simulink model "v01_aa_ri_8r4t11f_ver102"  (Simulink 7.6)
settings: Solver=ode45  StartTime=0.0  StopTime=128000  RelTol=1e-3
libraries: xbsIndex_r4 x13461, monroe_library x80, xbsReplacements_r4 x42, xps_library x36, casper_library_misc x35, simulink x23, dspsigops x16
(part 9 of 99)

== system v01_aa_ri_8r4t11f_ver102/adcsnap_b/bram_msb ==
  addr : Inport
  data_in : Inport  Port=2
  we : Inport  Port=3
  calc_add : Subsystem
  convert_addr : xbsIndex_r4/Convert  gui_display_data_type=Fixed-point  arith_type=Unsigned  n_bits=addr_width  bin_pt=0  float_type=Single  exp_bits=8  fraction_bits=24  quantization=Truncate  overflow=Wrap  en=off  latency=0  dbl_ovrd=off  pipeline=off  block_type=convert
      settings: xl_area=[0, 0, 0, 0, 0, 0, 0]
  convert_din : xbsIndex_r4/Convert  gui_display_data_type=Fixed-point  arith_type=Unsigned  n_bits=data_width  bin_pt=data_bin_pt  float_type=Single  exp_bits=8  fraction_bits=24  quantization=Truncate  overflow=Wrap  en=off  latency=0  dbl_ovrd=off  pipeline=off  block_type=convert
      settings: xl_area=[0, 0, 0, 0, 0, 0, 0]
  convert_din1 : xbsIndex_r4/Convert  gui_display_data_type=Fixed-point  arith_type=Unsigned  n_bits=data_width  bin_pt=data_bin_pt  float_type=Single  exp_bits=8  fraction_bits=24  quantization=Truncate  overflow=Wrap  en=off  latency=0  dbl_ovrd=off  pipeline=off  block_type=convert
      settings: xl_area=[0, 0, 0, 0, 0, 0, 0]
  convert_we : xbsIndex_r4/Convert  gui_display_data_type=Fixed-point  arith_type=Unsigned  n_bits=1  bin_pt=0  float_type=Single  exp_bits=8  fraction_bits=24  quantization=Truncate  overflow=Wrap  en=off  latency=0  dbl_ovrd=off  pipeline=off  block_type=convert
      settings: xl_area=[0, 0, 0, 0, 0, 0, 0]
  mem : Subsystem
  munge_in : Subsystem  AncestorBlock=casper_library_flow_control/munge  mask(munge){divisions=1, div_size=32, order=[0]}
      mask prompts: divisions "Number of divisions"; div_size "Division size (bits)"; order "Division packing order"
      mask help: Splits an input bus into the specified number of divisions and then recombines them in the order specified (most significant division = 0,least = number divisions-1)
      mask init:
      | munge_init(gcb, ...
      |     'divisions', divisions, ...
      |     'div_size', div_size, ...
      |     'order', order);
      settings: AttributesFormatString=|
      | [0]
      | 32 bits
  munge_out : Subsystem  AncestorBlock=casper_library_flow_control/munge  mask(munge){divisions=1, div_size=32, order=[0]}
      mask prompts: divisions "Number of divisions"; div_size "Division size (bits)"; order "Division packing order"
      mask help: Splits an input bus into the specified number of divisions and then recombines them in the order specified (most significant division = 0,least = number divisions-1)
      mask init:
      | munge_init(gcb, ...
      |     'divisions', divisions, ...
      |     'div_size', div_size, ...
      |     'order', order);
      settings: AttributesFormatString=|
      | [0]
      | 32 bits
  v01_aa_ri_8r4t11f_ver102_adcsnap_b_bram_msb_addr : xbsIndex_r4/Gateway Out  hdl_port=on  timing_constraint=None  locs_specified=off  LOCs={'A5','B9','A9','C1','A3','G6','E9','A6','A7','A8','B8','B1','B3','D9','B5','B4','B6','C2'}  block_type=gatewayout
      settings: infoedit="Gateway out block.  Converts Xilinx fixed point inputs into ouputs of type Simulink integer, double, or fixed point.<P><P>Hardware notes:  In hardware these blocks become top level output ports or are discarded, depending on how they are configured."  xl_area=[0, 0, 0, 0, 0, 0, 0]  sginterface={'Nallatech'=>{'xdspkit'=>{'non_memory_mapped_port'=>'uprev_Shared_BRAM_addr'}},'Xilinx'=>{'jtaghwcosim'=>{'non_memory_mapped_port'=>'uprev_Shared_BRAM_addr'},'xdspkit'=>{'non_memory_mapped_port'=>'uprev_Shared_BRAM_addr'}}}
  v01_aa_ri_8r4t11f_ver102_adcsnap_b_bram_msb_data_in : xbsIndex_r4/Gateway Out  hdl_port=on  timing_constraint=None  locs_specified=off  LOCs={'B21','B22','A22','B23','B19','C19','A20','A21','A23','B24','A24','A25','E18','D18','C18','B18'}  block_type=gatewayout
      settings: infoedit="Gateway out block.  Converts Xilinx fixed point inputs into ouputs of type Simulink integer, double, or fixed point.<P><P>Hardware notes:  In hardware these blocks become top level output ports or are discarded, depending on how they are configured."  xl_area=[0, 0, 0, 0, 0, 0, 0]  sginterface={'Nallatech'=>{'xdspkit'=>{'non_memory_mapped_port'=>'uprev_Shared_BRAM_data_in'}},'Xilinx'=>{'jtaghwcosim'=>{'non_memory_mapped_port'=>'uprev_Shared_BRAM_data_in'},'xdspkit'=>{'non_memory_mapped_port'=>'uprev_Shared_BRAM_data_in'}}}
  v01_aa_ri_8r4t11f_ver102_adcsnap_b_bram_msb_data_out : xbsIndex_r4/Gateway In  gui_display_data_type=Fixed-point  arith_type=Unsigned  n_bits=data_width  bin_pt=data_bin_pt  preci_type=Single  exp_width=8  frac_width=24  quantization=Truncate  overflow=Wrap  period=sample_rate  dbl_ovrd=off  timing_constraint=None  locs_specified=off  LOCs={}  hdl_port=on  block_type=gatewayin
      settings: infoedit="Gateway in block.  Converts inputs of type Simulink integer, double and fixed point to  Xilinx fixed point type.<br><br>Hardware notes:  In hardware these blocks become top level input ports."  xl_area=[0, 0, 0, 0, 0, 0, 0]
  v01_aa_ri_8r4t11f_ver102_adcsnap_b_bram_msb_we : xbsIndex_r4/Gateway Out  hdl_port=on  timing_constraint=None  locs_specified=off  LOCs={'A14'}  block_type=gatewayout
      settings: infoedit="Gateway out block.  Converts Xilinx fixed point inputs into ouputs of type Simulink integer, double, or fixed point.<P><P>Hardware notes:  In hardware these blocks become top level output ports or are discarded, depending on how they are configured."  xl_area=[0, 0, 0, 0, 0, 0, 0]
  data_out : Outport
  wires:
  mem/1(Out1) -> v01_aa_ri_8r4t11f_ver102_adcsnap_b_bram_msb_data_out/1
  data_in/1 -> convert_din/1
  v01_aa_ri_8r4t11f_ver102_adcsnap_b_bram_msb_data_out/1 -> munge_out/1(din)
  addr/1 -> calc_add/1(in)
  we/1 -> convert_we/1
  convert_we/1 -> v01_aa_ri_8r4t11f_ver102_adcsnap_b_bram_msb_we/1
  v01_aa_ri_8r4t11f_ver102_adcsnap_b_bram_msb_addr/1 -> mem/1(In1)
  v01_aa_ri_8r4t11f_ver102_adcsnap_b_bram_msb_data_in/1 -> mem/2(In2)
  v01_aa_ri_8r4t11f_ver102_adcsnap_b_bram_msb_we/1 -> mem/3(In3)
  munge_in/1(dout) -> convert_din1/1
  munge_out/1(dout) -> data_out/1
  calc_add/1(out) -> convert_addr/1
  convert_addr/1 -> v01_aa_ri_8r4t11f_ver102_adcsnap_b_bram_msb_addr/1
  convert_din1/1 -> v01_aa_ri_8r4t11f_ver102_adcsnap_b_bram_msb_data_in/1
  convert_din/1 -> munge_in/1(din)

== system v01_aa_ri_8r4t11f_ver102/fft_wideband_real/fft_biplex_real_4x0 ==
  sync : Inport
  shift : Inport  Port=2
  pol1 : Inport  Port=3
  pol2 : Inport  Port=4
  pol3 : Inport  Port=5
  pol4 : Inport  Port=6
  bi_real_unscr_4x : Subsystem
  biplex_core : Subsystem
      settings: AttributesFormatString=|
      | 8 stages
      | reduce logic
      | Virtex5
  ri_to_c_pol1 : Subsystem
  ri_to_c_pol2 : Subsystem
  sync_out : Outport
  pol1_out : Outport  Port=2
  pol2_out : Outport  Port=3
  pol3_out : Outport  Port=4
  pol4_out : Outport  Port=5
  of : Outport  Port=6
  wires:
  bi_real_unscr_4x/5(pol4_out) -> pol4_out/1
  bi_real_unscr_4x/4(pol3_out) -> pol3_out/1
  bi_real_unscr_4x/3(pol2_out) -> pol2_out/1
  bi_real_unscr_4x/2(pol1_out) -> pol1_out/1
  bi_real_unscr_4x/1(sync_out) -> sync_out/1
  biplex_core/3(out2) -> bi_real_unscr_4x/3(odd)
  biplex_core/2(out1) -> bi_real_unscr_4x/2(even)
  biplex_core/1(sync_out) -> bi_real_unscr_4x/1(sync)
  biplex_core/4(of) -> of/1
  ri_to_c_pol2/1(c) -> biplex_core/4(pol2)
  ri_to_c_pol1/1(c) -> biplex_core/3(pol1)
  shift/1 -> biplex_core/2(shift)
  sync/1 -> biplex_core/1(sync)
  pol4/1 -> ri_to_c_pol2/2(im)
  pol3/1 -> ri_to_c_pol2/1(re)
  pol2/1 -> ri_to_c_pol1/2(im)
  pol1/1 -> ri_to_c_pol1/1(re)

== system v01_aa_ri_8r4t11f_ver102/fft_wideband_real/fft_biplex_real_4x1 ==
  sync : Inport
  shift : Inport  Port=2
  pol1 : Inport  Port=3
  pol2 : Inport  Port=4
  pol3 : Inport  Port=5
  pol4 : Inport  Port=6
  bi_real_unscr_4x : Subsystem
  biplex_core : Subsystem
      settings: AttributesFormatString=|
      | 8 stages
      | reduce logic
      | Virtex5
  ri_to_c_pol1 : Subsystem
  ri_to_c_pol2 : Subsystem
  sync_out : Outport
  pol1_out : Outport  Port=2
  pol2_out : Outport  Port=3
  pol3_out : Outport  Port=4
  pol4_out : Outport  Port=5
  of : Outport  Port=6
  wires:
  bi_real_unscr_4x/5(pol4_out) -> pol4_out/1
  bi_real_unscr_4x/4(pol3_out) -> pol3_out/1
  bi_real_unscr_4x/3(pol2_out) -> pol2_out/1
  bi_real_unscr_4x/2(pol1_out) -> pol1_out/1
  bi_real_unscr_4x/1(sync_out) -> sync_out/1
  biplex_core/3(out2) -> bi_real_unscr_4x/3(odd)
  biplex_core/2(out1) -> bi_real_unscr_4x/2(even)
  biplex_core/1(sync_out) -> bi_real_unscr_4x/1(sync)
  biplex_core/4(of) -> of/1
  ri_to_c_pol2/1(c) -> biplex_core/4(pol2)
  ri_to_c_pol1/1(c) -> biplex_core/3(pol1)
  shift/1 -> biplex_core/2(shift)
  sync/1 -> biplex_core/1(sync)
  pol4/1 -> ri_to_c_pol2/2(im)
  pol3/1 -> ri_to_c_pol2/1(re)
  pol2/1 -> ri_to_c_pol1/2(im)
  pol1/1 -> ri_to_c_pol1/1(re)

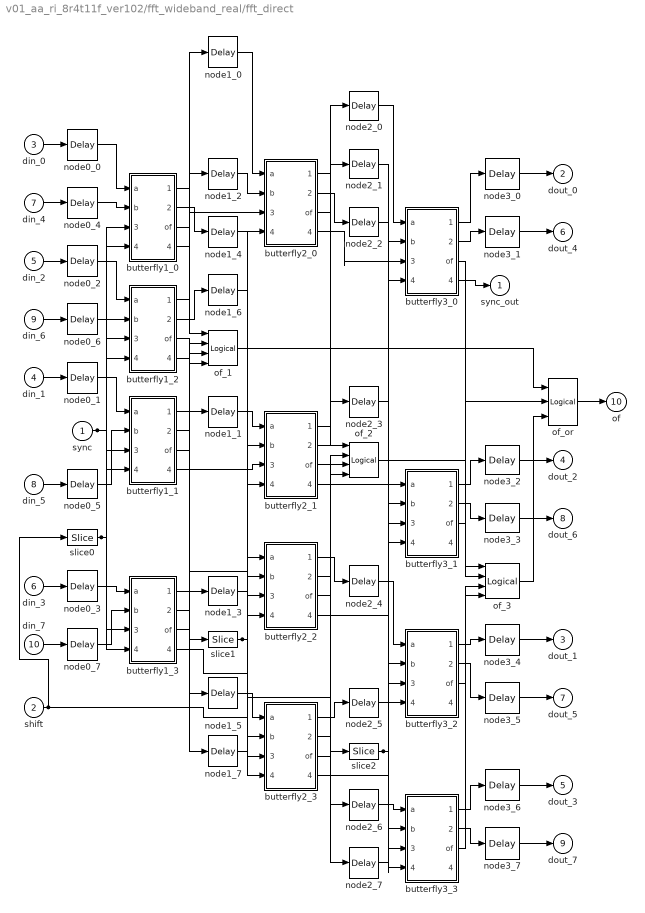
== system v01_aa_ri_8r4t11f_ver102/fft_wideband_real/fft_direct ==
  sync : Inport
  shift : Inport  Port=2
  din_0 : Inport  Port=3
  din_1 : Inport  Port=4
  din_2 : Inport  Port=5
  din_3 : Inport  Port=6
  din_4 : Inport  Port=7
  din_5 : Inport  Port=8
  din_6 : Inport  Port=9
  din_7 : Inport  Port=10
  butterfly1_0 : Subsystem
      settings: AttributesFormatString=|
      | twiddle_general_4mult
      | coeffs in BRAM
      | Bit growth:0
  butterfly1_1 : Subsystem
      settings: AttributesFormatString=|
      | twiddle_general_4mult
      | coeffs in BRAM
      | Bit growth:0
  butterfly1_2 : Subsystem
      settings: AttributesFormatString=|
      | twiddle_general_4mult
      | coeffs in BRAM
      | Bit growth:0
  butterfly1_3 : Subsystem
      settings: AttributesFormatString=|
      | twiddle_general_4mult
      | coeffs in BRAM
      | Bit growth:0
  butterfly2_0 : Subsystem
      settings: AttributesFormatString=|
      | twiddle_general_4mult
      | coeffs in BRAM
      | Bit growth:0
  butterfly2_1 : Subsystem
      settings: AttributesFormatString=|
      | twiddle_general_4mult
      | coeffs in BRAM
      | Bit growth:0
  butterfly2_2 : Subsystem
      settings: AttributesFormatString=|
      | twiddle_general_4mult
      | coeffs in BRAM
      | Bit growth:0
  butterfly2_3 : Subsystem
      settings: AttributesFormatString=|
      | twiddle_general_4mult
      | coeffs in BRAM
      | Bit growth:0
  butterfly3_0 : Subsystem
      settings: AttributesFormatString=|
      | twiddle_general_4mult
      | coeffs in BRAM
      | Bit growth:0
  butterfly3_1 : Subsystem
      settings: AttributesFormatString=|
      | twiddle_general_4mult
      | coeffs in BRAM
      | Bit growth:0
  butterfly3_2 : Subsystem
      settings: AttributesFormatString=|
      | twiddle_general_4mult
      | coeffs in BRAM
      | Bit growth:0
  butterfly3_3 : Subsystem
      settings: AttributesFormatString=|
      | twiddle_general_4mult
      | coeffs in BRAM
      | Bit growth:0
  node0_{0..7} (8 alike) : xbsIndex_r4/Delay  rst=off  infoeditControl=Selection of Reset will increase slice count due to use of real FFs and instead of SRLs  en=off  latency=0  dbl_ovrd=off  reg_retiming=off  block_type=delay
  node1_{0..7} (8 alike) : xbsIndex_r4/Delay  rst=off  infoeditControl=Selection of Reset will increase slice count due to use of real FFs and instead of SRLs  en=off  latency=0  dbl_ovrd=off  reg_retiming=off  block_type=delay
  node2_{0..7} (8 alike) : xbsIndex_r4/Delay  rst=off  infoeditControl=Selection of Reset will increase slice count due to use of real FFs and instead of SRLs  en=off  latency=0  dbl_ovrd=off  reg_retiming=off  block_type=delay
  node3_{0..7} (8 alike) : xbsIndex_r4/Delay  rst=off  infoeditControl=Selection of Reset will increase slice count due to use of real FFs and instead of SRLs  en=off  latency=0  dbl_ovrd=off  reg_retiming=off  block_type=delay
  of_1 : xbsIndex_r4/Logical  logical_function=OR  inputs=4  en=off  latency=1  precision=Full  arith_type=Unsigned  n_bits=16  bin_pt=0  align_bp=on  dbl_ovrd=off  block_type=logical
  of_2 : xbsIndex_r4/Logical  logical_function=OR  inputs=4  en=off  latency=1  precision=Full  arith_type=Unsigned  n_bits=16  bin_pt=0  align_bp=on  dbl_ovrd=off  block_type=logical
  of_3 : xbsIndex_r4/Logical  logical_function=OR  inputs=4  en=off  latency=1  precision=Full  arith_type=Unsigned  n_bits=16  bin_pt=0  align_bp=on  dbl_ovrd=off  block_type=logical
  of_or : xbsIndex_r4/Logical  logical_function=OR  inputs=3  en=off  latency=0  precision=Full  arith_type=Unsigned  n_bits=16  bin_pt=0  align_bp=on  dbl_ovrd=off  block_type=logical
  slice0 : xbsIndex_r4/Slice  nbits=1  boolean_output=on  mode=Lower Bit Location + Width  bit1=0  base1=MSB of Input  bit0=0  base0=LSB of Input  dbl_ovrd=off  block_type=slice
  slice1 : xbsIndex_r4/Slice  nbits=1  boolean_output=on  mode=Lower Bit Location + Width  bit1=0  base1=MSB of Input  bit0=1  base0=LSB of Input  dbl_ovrd=off  block_type=slice
  slice2 : xbsIndex_r4/Slice  nbits=1  boolean_output=on  mode=Lower Bit Location + Width  bit1=0  base1=MSB of Input  bit0=2  base0=LSB of Input  dbl_ovrd=off  block_type=slice
  sync_out : Outport
  dout_0 : Outport  Port=2
  dout_1 : Outport  Port=3
  dout_2 : Outport  Port=4
  dout_3 : Outport  Port=5
  dout_4 : Outport  Port=6
  dout_5 : Outport  Port=7
  dout_6 : Outport  Port=8
  dout_7 : Outport  Port=9
  of : Outport  Port=10
  wires:
  shift/1 -> slice2/1, slice1/1, slice0/1
  sync/1 -> butterfly1_3/3(sync), butterfly1_2/3(sync), butterfly1_1/3(sync), butterfly1_0/3(sync)
  slice0/1 -> butterfly1_3/4(shift), butterfly1_2/4(shift), butterfly1_1/4(shift), butterfly1_0/4(shift)
  slice1/1 -> butterfly2_3/4(shift), butterfly2_2/4(shift), butterfly2_1/4(shift), butterfly2_0/4(shift)
  slice2/1 -> butterfly3_3/4(shift), butterfly3_2/4(shift), butterfly3_1/4(shift), butterfly3_0/4(shift)
  butterfly3_0/4(sync_out) -> sync_out/1
  of_or/1 -> of/1
  of_3/1 -> of_or/3
  of_2/1 -> of_or/2
  of_1/1 -> of_or/1
  butterfly3_3/3(of) -> of_3/4
  butterfly3_2/3(of) -> of_3/3
  butterfly3_1/3(of) -> of_3/2
  butterfly3_0/3(of) -> of_3/1
  butterfly3_3/2(a-bw) -> node3_7/1
  butterfly3_3/1(a+bw) -> node3_6/1
  butterfly2_3/4(sync_out) -> butterfly3_3/3(sync)
  node2_7/1 -> butterfly3_3/2(b)
  node2_6/1 -> butterfly3_3/1(a)
  butterfly3_2/2(a-bw) -> node3_5/1
  butterfly3_2/1(a+bw) -> node3_4/1
  butterfly2_2/4(sync_out) -> butterfly3_2/3(sync)
  node2_5/1 -> butterfly3_2/2(b)
  node2_4/1 -> butterfly3_2/1(a)
  butterfly3_1/2(a-bw) -> node3_3/1
  butterfly3_1/1(a+bw) -> node3_2/1
  butterfly2_1/4(sync_out) -> butterfly3_1/3(sync)
  node2_3/1 -> butterfly3_1/2(b)
  node2_2/1 -> butterfly3_1/1(a)
  butterfly3_0/2(a-bw) -> node3_1/1
  butterfly3_0/1(a+bw) -> node3_0/1
  butterfly2_0/4(sync_out) -> butterfly3_0/3(sync)
  node2_1/1 -> butterfly3_0/2(b)
  node2_0/1 -> butterfly3_0/1(a)
  butterfly2_3/3(of) -> of_2/4
  butterfly2_2/3(of) -> of_2/3
  butterfly2_1/3(of) -> of_2/2
  butterfly2_0/3(of) -> of_2/1
  butterfly2_3/2(a-bw) -> node2_7/1
  butterfly2_3/1(a+bw) -> node2_5/1
  butterfly1_3/4(sync_out) -> butterfly2_3/3(sync)
  node1_7/1 -> butterfly2_3/2(b)
  node1_5/1 -> butterfly2_3/1(a)
  butterfly2_2/2(a-bw) -> node2_6/1
  butterfly2_2/1(a+bw) -> node2_4/1
  butterfly1_2/4(sync_out) -> butterfly2_2/3(sync)
  node1_6/1 -> butterfly2_2/2(b)
  node1_4/1 -> butterfly2_2/1(a)
  butterfly2_1/2(a-bw) -> node2_3/1
  butterfly2_1/1(a+bw) -> node2_1/1
  butterfly1_1/4(sync_out) -> butterfly2_1/3(sync)
  node1_3/1 -> butterfly2_1/2(b)
  node1_1/1 -> butterfly2_1/1(a)
  butterfly2_0/2(a-bw) -> node2_2/1
  butterfly2_0/1(a+bw) -> node2_0/1
  butterfly1_0/4(sync_out) -> butterfly2_0/3(sync)
  node1_2/1 -> butterfly2_0/2(b)
  node1_0/1 -> butterfly2_0/1(a)
  butterfly1_3/3(of) -> of_1/4
  butterfly1_2/3(of) -> of_1/3
  butterfly1_1/3(of) -> of_1/2
  butterfly1_0/3(of) -> of_1/1
  butterfly1_3/2(a-bw) -> node1_7/1
  butterfly1_3/1(a+bw) -> node1_3/1
  node0_7/1 -> butterfly1_3/2(b)
  node0_3/1 -> butterfly1_3/1(a)
  butterfly1_2/2(a-bw) -> node1_6/1
  butterfly1_2/1(a+bw) -> node1_2/1
  node0_6/1 -> butterfly1_2/2(b)
  node0_2/1 -> butterfly1_2/1(a)
  butterfly1_1/2(a-bw) -> node1_5/1
  butterfly1_1/1(a+bw) -> node1_1/1
  node0_5/1 -> butterfly1_1/2(b)
  node0_1/1 -> butterfly1_1/1(a)
  butterfly1_0/2(a-bw) -> node1_4/1
  butterfly1_0/1(a+bw) -> node1_0/1
  node0_4/1 -> butterfly1_0/2(b)
  node0_0/1 -> butterfly1_0/1(a)
  node3_7/1 -> dout_7/1
  node3_6/1 -> dout_3/1
  node3_5/1 -> dout_5/1
  node3_4/1 -> dout_1/1
  node3_3/1 -> dout_6/1
  node3_2/1 -> dout_2/1
  node3_1/1 -> dout_4/1
  node3_0/1 -> dout_0/1
  din_7/1 -> node0_7/1
  din_6/1 -> node0_6/1
  din_5/1 -> node0_5/1
  din_4/1 -> node0_4/1
  din_3/1 -> node0_3/1
  din_2/1 -> node0_2/1
  din_1/1 -> node0_1/1
  din_0/1 -> node0_0/1

== system v01_aa_ri_8r4t11f_ver102/fft_wideband_real/fft_unscrambler ==
  sync : Inport
  In1 : Inport  Port=2
  In2 : Inport  Port=3
  In3 : Inport  Port=4
  In4 : Inport  Port=5
  const : xbsIndex_r4/Constant  const=1  gui_display_data_type=Fixed-point  arith_type=Boolean  n_bits=1  bin_pt=0  preci_type=Single  exp_width=8  frac_width=24  explicit_period=on  period=1  equ=P=C  opselect=C  inp2=PCIN>>17  opr=+  inp1=P  carry=CIN  dbl_ovrd=off  block_type=constant
  reorder1 : Subsystem
      settings: AttributesFormatString=order=4
  square_transposer1 : Subsystem
      settings: AttributesFormatString=n_inputs=2
  sync_out : Outport
  Out1 : Outport  Port=2
  Out2 : Outport  Port=3
  Out3 : Outport  Port=4
  Out4 : Outport  Port=5
  wires:
  square_transposer1/5(Out4) -> reorder1/6(din3)  "xlsub2_reorder_In4"
  square_transposer1/4(Out3) -> reorder1/5(din2)  "xlsub2_reorder_In3"
  square_transposer1/3(Out2) -> reorder1/4(din1)  "xlsub2_reorder_In2"
  square_transposer1/2(Out1) -> reorder1/3(din0)  "xlsub2_reorder_In1"
  square_transposer1/1(sync_out) -> reorder1/1(sync)
  In4/1 -> square_transposer1/5(In4)
  In3/1 -> square_transposer1/4(In3)
  In2/1 -> square_transposer1/3(In2)
  In1/1 -> square_transposer1/2(In1)
  sync/1 -> square_transposer1/1(sync)
  reorder1/6(dout3) -> Out4/1
  reorder1/5(dout2) -> Out3/1
  reorder1/4(dout1) -> Out2/1
  reorder1/3(dout0) -> Out1/1
  reorder1/1(sync_out) -> sync_out/1
  const/1 -> reorder1/2(en)

== system v01_aa_ri_8r4t11f_ver102/fft_wideband_real/in_del_4x0pol1 ==
  d : Inport
  q : Outport
  wires:
  d/1 -> q/1

== system v01_aa_ri_8r4t11f_ver102/fft_wideband_real/in_del_4x0pol2 ==
  d : Inport
  q : Outport
  wires:
  d/1 -> q/1

== system v01_aa_ri_8r4t11f_ver102/fft_wideband_real/in_del_4x0pol3 ==
  d : Inport
  q : Outport
  wires:
  d/1 -> q/1

== system v01_aa_ri_8r4t11f_ver102/fft_wideband_real/in_del_4x0pol4 ==
  d : Inport
  q : Outport
  wires:
  d/1 -> q/1

== system v01_aa_ri_8r4t11f_ver102/fft_wideband_real/in_del_4x1pol1 ==
  d : Inport
  q : Outport
  wires:
  d/1 -> q/1

== system v01_aa_ri_8r4t11f_ver102/fft_wideband_real/in_del_4x1pol2 ==
  d : Inport
  q : Outport
  wires:
  d/1 -> q/1

== system v01_aa_ri_8r4t11f_ver102/fft_wideband_real/in_del_4x1pol3 ==
  d : Inport
  q : Outport
  wires:
  d/1 -> q/1

== system v01_aa_ri_8r4t11f_ver102/fft_wideband_real/in_del_4x1pol4 ==
  d : Inport
  q : Outport
  wires:
  d/1 -> q/1

== system v01_aa_ri_8r4t11f_ver102/fft_wideband_real/in_del_sync_4x0 ==
  d : Inport
  q : Outport
  wires:
  d/1 -> q/1

== system v01_aa_ri_8r4t11f_ver102/fft_wideband_real/in_del_sync_4x1 ==
  d : Inport
  q : Outport
  wires:
  d/1 -> q/1

== system v01_aa_ri_8r4t11f_ver102/fft_wideband_real/out_del_4x0pol1 ==
  d : Inport
  q : Outport
  wires:
  d/1 -> q/1

== system v01_aa_ri_8r4t11f_ver102/fft_wideband_real/out_del_4x0pol2 ==
  d : Inport
  q : Outport
  wires:
  d/1 -> q/1

== system v01_aa_ri_8r4t11f_ver102/fft_wideband_real/out_del_4x0pol3 ==
  d : Inport
  q : Outport
  wires:
  d/1 -> q/1

== system v01_aa_ri_8r4t11f_ver102/fft_wideband_real/out_del_4x0pol4 ==
  d : Inport
  q : Outport
  wires:
  d/1 -> q/1

== system v01_aa_ri_8r4t11f_ver102/fft_wideband_real/out_del_4x1pol1 ==
  d : Inport
  q : Outport
  wires:
  d/1 -> q/1

== system v01_aa_ri_8r4t11f_ver102/fft_wideband_real/out_del_4x1pol2 ==
  d : Inport
  q : Outport
  wires:
  d/1 -> q/1

== system v01_aa_ri_8r4t11f_ver102/fft_wideband_real/out_del_4x1pol3 ==
  d : Inport
  q : Outport
  wires:
  d/1 -> q/1

== system v01_aa_ri_8r4t11f_ver102/fft_wideband_real/out_del_4x1pol4 ==
  d : Inport
  q : Outport
  wires:
  d/1 -> q/1

== system v01_aa_ri_8r4t11f_ver102/fft_wideband_real/out_del_sync_4x0 ==
  d : Inport
  q : Outport
  wires:
  d/1 -> q/1

== system v01_aa_ri_8r4t11f_ver102/fft_wideband_real1/fft_biplex_real_4x0 ==
  sync : Inport
  shift : Inport  Port=2
  pol1 : Inport  Port=3
  pol2 : Inport  Port=4
  pol3 : Inport  Port=5
  pol4 : Inport  Port=6
  bi_real_unscr_4x : Subsystem
  biplex_core : Subsystem
      settings: AttributesFormatString=|
      | 8 stages
      | reduce logic
      | Virtex5
  ri_to_c_pol1 : Subsystem
  ri_to_c_pol2 : Subsystem
  sync_out : Outport
  pol1_out : Outport  Port=2
  pol2_out : Outport  Port=3
  pol3_out : Outport  Port=4
  pol4_out : Outport  Port=5
  of : Outport  Port=6
  wires:
  bi_real_unscr_4x/5(pol4_out) -> pol4_out/1
  bi_real_unscr_4x/4(pol3_out) -> pol3_out/1
  bi_real_unscr_4x/3(pol2_out) -> pol2_out/1
  bi_real_unscr_4x/2(pol1_out) -> pol1_out/1
  bi_real_unscr_4x/1(sync_out) -> sync_out/1
  biplex_core/3(out2) -> bi_real_unscr_4x/3(odd)
  biplex_core/2(out1) -> bi_real_unscr_4x/2(even)
  biplex_core/1(sync_out) -> bi_real_unscr_4x/1(sync)
  biplex_core/4(of) -> of/1
  ri_to_c_pol2/1(c) -> biplex_core/4(pol2)
  ri_to_c_pol1/1(c) -> biplex_core/3(pol1)
  shift/1 -> biplex_core/2(shift)
  sync/1 -> biplex_core/1(sync)
  pol4/1 -> ri_to_c_pol2/2(im)
  pol3/1 -> ri_to_c_pol2/1(re)
  pol2/1 -> ri_to_c_pol1/2(im)
  pol1/1 -> ri_to_c_pol1/1(re)

== system v01_aa_ri_8r4t11f_ver102/fft_wideband_real1/fft_biplex_real_4x1 ==
  sync : Inport
  shift : Inport  Port=2
  pol1 : Inport  Port=3
  pol2 : Inport  Port=4
  pol3 : Inport  Port=5
  pol4 : Inport  Port=6
  bi_real_unscr_4x : Subsystem
  biplex_core : Subsystem
      settings: AttributesFormatString=|
      | 8 stages
      | reduce logic
      | Virtex5
  ri_to_c_pol1 : Subsystem
  ri_to_c_pol2 : Subsystem
  sync_out : Outport
  pol1_out : Outport  Port=2
  pol2_out : Outport  Port=3
  pol3_out : Outport  Port=4
  pol4_out : Outport  Port=5
  of : Outport  Port=6
  wires:
  bi_real_unscr_4x/5(pol4_out) -> pol4_out/1
  bi_real_unscr_4x/4(pol3_out) -> pol3_out/1
  bi_real_unscr_4x/3(pol2_out) -> pol2_out/1
  bi_real_unscr_4x/2(pol1_out) -> pol1_out/1
  bi_real_unscr_4x/1(sync_out) -> sync_out/1
  biplex_core/3(out2) -> bi_real_unscr_4x/3(odd)
  biplex_core/2(out1) -> bi_real_unscr_4x/2(even)
  biplex_core/1(sync_out) -> bi_real_unscr_4x/1(sync)
  biplex_core/4(of) -> of/1
  ri_to_c_pol2/1(c) -> biplex_core/4(pol2)
  ri_to_c_pol1/1(c) -> biplex_core/3(pol1)
  shift/1 -> biplex_core/2(shift)
  sync/1 -> biplex_core/1(sync)
  pol4/1 -> ri_to_c_pol2/2(im)
  pol3/1 -> ri_to_c_pol2/1(re)
  pol2/1 -> ri_to_c_pol1/2(im)
  pol1/1 -> ri_to_c_pol1/1(re)

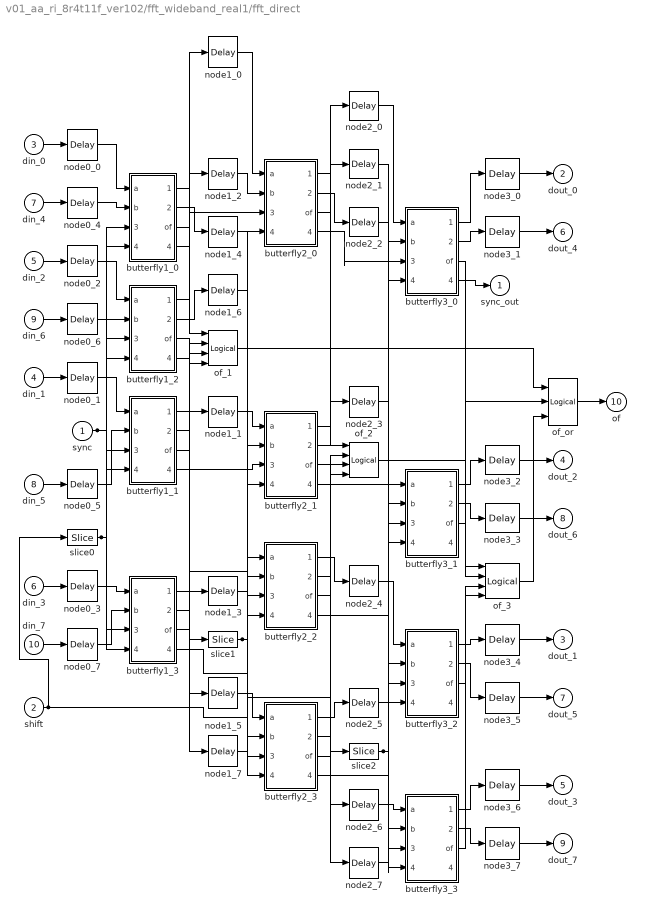
== system v01_aa_ri_8r4t11f_ver102/fft_wideband_real1/fft_direct ==
  sync : Inport
  shift : Inport  Port=2
  din_0 : Inport  Port=3
  din_1 : Inport  Port=4
  din_2 : Inport  Port=5
  din_3 : Inport  Port=6
  din_4 : Inport  Port=7
  din_5 : Inport  Port=8
  din_6 : Inport  Port=9
  din_7 : Inport  Port=10
  butterfly1_0 : Subsystem
      settings: AttributesFormatString=|
      | twiddle_general_4mult
      | coeffs in BRAM
      | Bit growth:0
  butterfly1_1 : Subsystem
      settings: AttributesFormatString=|
      | twiddle_general_4mult
      | coeffs in BRAM
      | Bit growth:0
  butterfly1_2 : Subsystem
      settings: AttributesFormatString=|
      | twiddle_general_4mult
      | coeffs in BRAM
      | Bit growth:0
  butterfly1_3 : Subsystem
      settings: AttributesFormatString=|
      | twiddle_general_4mult
      | coeffs in BRAM
      | Bit growth:0
  butterfly2_0 : Subsystem
      settings: AttributesFormatString=|
      | twiddle_general_4mult
      | coeffs in BRAM
      | Bit growth:0
  butterfly2_1 : Subsystem
      settings: AttributesFormatString=|
      | twiddle_general_4mult
      | coeffs in BRAM
      | Bit growth:0
  butterfly2_2 : Subsystem
      settings: AttributesFormatString=|
      | twiddle_general_4mult
      | coeffs in BRAM
      | Bit growth:0
  butterfly2_3 : Subsystem
      settings: AttributesFormatString=|
      | twiddle_general_4mult
      | coeffs in BRAM
      | Bit growth:0
  butterfly3_0 : Subsystem
      settings: AttributesFormatString=|
      | twiddle_general_4mult
      | coeffs in BRAM
      | Bit growth:0
  butterfly3_1 : Subsystem
      settings: AttributesFormatString=|
      | twiddle_general_4mult
      | coeffs in BRAM
      | Bit growth:0
  butterfly3_2 : Subsystem
      settings: AttributesFormatString=|
      | twiddle_general_4mult
      | coeffs in BRAM
      | Bit growth:0
  butterfly3_3 : Subsystem
      settings: AttributesFormatString=|
      | twiddle_general_4mult
      | coeffs in BRAM
      | Bit growth:0
  node0_{0..7} (8 alike) : xbsIndex_r4/Delay  rst=off  infoeditControl=Selection of Reset will increase slice count due to use of real FFs and instead of SRLs  en=off  latency=0  dbl_ovrd=off  reg_retiming=off  block_type=delay
  node1_{0..7} (8 alike) : xbsIndex_r4/Delay  rst=off  infoeditControl=Selection of Reset will increase slice count due to use of real FFs and instead of SRLs  en=off  latency=0  dbl_ovrd=off  reg_retiming=off  block_type=delay
  node2_{0..7} (8 alike) : xbsIndex_r4/Delay  rst=off  infoeditControl=Selection of Reset will increase slice count due to use of real FFs and instead of SRLs  en=off  latency=0  dbl_ovrd=off  reg_retiming=off  block_type=delay
  node3_{0..7} (8 alike) : xbsIndex_r4/Delay  rst=off  infoeditControl=Selection of Reset will increase slice count due to use of real FFs and instead of SRLs  en=off  latency=0  dbl_ovrd=off  reg_retiming=off  block_type=delay
  of_1 : xbsIndex_r4/Logical  logical_function=OR  inputs=4  en=off  latency=1  precision=Full  arith_type=Unsigned  n_bits=16  bin_pt=0  align_bp=on  dbl_ovrd=off  block_type=logical
  of_2 : xbsIndex_r4/Logical  logical_function=OR  inputs=4  en=off  latency=1  precision=Full  arith_type=Unsigned  n_bits=16  bin_pt=0  align_bp=on  dbl_ovrd=off  block_type=logical
  of_3 : xbsIndex_r4/Logical  logical_function=OR  inputs=4  en=off  latency=1  precision=Full  arith_type=Unsigned  n_bits=16  bin_pt=0  align_bp=on  dbl_ovrd=off  block_type=logical
  of_or : xbsIndex_r4/Logical  logical_function=OR  inputs=3  en=off  latency=0  precision=Full  arith_type=Unsigned  n_bits=16  bin_pt=0  align_bp=on  dbl_ovrd=off  block_type=logical
  slice0 : xbsIndex_r4/Slice  nbits=1  boolean_output=on  mode=Lower Bit Location + Width  bit1=0  base1=MSB of Input  bit0=0  base0=LSB of Input  dbl_ovrd=off  block_type=slice
  slice1 : xbsIndex_r4/Slice  nbits=1  boolean_output=on  mode=Lower Bit Location + Width  bit1=0  base1=MSB of Input  bit0=1  base0=LSB of Input  dbl_ovrd=off  block_type=slice
  slice2 : xbsIndex_r4/Slice  nbits=1  boolean_output=on  mode=Lower Bit Location + Width  bit1=0  base1=MSB of Input  bit0=2  base0=LSB of Input  dbl_ovrd=off  block_type=slice
  sync_out : Outport
  dout_0 : Outport  Port=2
  dout_1 : Outport  Port=3
  dout_2 : Outport  Port=4
  dout_3 : Outport  Port=5
  dout_4 : Outport  Port=6
  dout_5 : Outport  Port=7
  dout_6 : Outport  Port=8
  dout_7 : Outport  Port=9
  of : Outport  Port=10
  wires:
  shift/1 -> slice2/1, slice1/1, slice0/1
  sync/1 -> butterfly1_3/3(sync), butterfly1_2/3(sync), butterfly1_1/3(sync), butterfly1_0/3(sync)
  slice0/1 -> butterfly1_3/4(shift), butterfly1_2/4(shift), butterfly1_1/4(shift), butterfly1_0/4(shift)
  slice1/1 -> butterfly2_3/4(shift), butterfly2_2/4(shift), butterfly2_1/4(shift), butterfly2_0/4(shift)
  slice2/1 -> butterfly3_3/4(shift), butterfly3_2/4(shift), butterfly3_1/4(shift), butterfly3_0/4(shift)
  butterfly3_0/4(sync_out) -> sync_out/1
  of_or/1 -> of/1
  of_3/1 -> of_or/3
  of_2/1 -> of_or/2
  of_1/1 -> of_or/1
  butterfly3_3/3(of) -> of_3/4
  butterfly3_2/3(of) -> of_3/3
  butterfly3_1/3(of) -> of_3/2
  butterfly3_0/3(of) -> of_3/1
  butterfly3_3/2(a-bw) -> node3_7/1
  butterfly3_3/1(a+bw) -> node3_6/1
  butterfly2_3/4(sync_out) -> butterfly3_3/3(sync)
  node2_7/1 -> butterfly3_3/2(b)
  node2_6/1 -> butterfly3_3/1(a)
  butterfly3_2/2(a-bw) -> node3_5/1
  butterfly3_2/1(a+bw) -> node3_4/1
  butterfly2_2/4(sync_out) -> butterfly3_2/3(sync)
  node2_5/1 -> butterfly3_2/2(b)
  node2_4/1 -> butterfly3_2/1(a)
  butterfly3_1/2(a-bw) -> node3_3/1
  butterfly3_1/1(a+bw) -> node3_2/1
  butterfly2_1/4(sync_out) -> butterfly3_1/3(sync)
  node2_3/1 -> butterfly3_1/2(b)
  node2_2/1 -> butterfly3_1/1(a)
  butterfly3_0/2(a-bw) -> node3_1/1
  butterfly3_0/1(a+bw) -> node3_0/1
  butterfly2_0/4(sync_out) -> butterfly3_0/3(sync)
  node2_1/1 -> butterfly3_0/2(b)
  node2_0/1 -> butterfly3_0/1(a)
  butterfly2_3/3(of) -> of_2/4
  butterfly2_2/3(of) -> of_2/3
  butterfly2_1/3(of) -> of_2/2
  butterfly2_0/3(of) -> of_2/1
  butterfly2_3/2(a-bw) -> node2_7/1
  butterfly2_3/1(a+bw) -> node2_5/1
  butterfly1_3/4(sync_out) -> butterfly2_3/3(sync)
  node1_7/1 -> butterfly2_3/2(b)
  node1_5/1 -> butterfly2_3/1(a)
  butterfly2_2/2(a-bw) -> node2_6/1
  butterfly2_2/1(a+bw) -> node2_4/1
  butterfly1_2/4(sync_out) -> butterfly2_2/3(sync)
  node1_6/1 -> butterfly2_2/2(b)
  node1_4/1 -> butterfly2_2/1(a)
  butterfly2_1/2(a-bw) -> node2_3/1
  butterfly2_1/1(a+bw) -> node2_1/1
  butterfly1_1/4(sync_out) -> butterfly2_1/3(sync)
  node1_3/1 -> butterfly2_1/2(b)
  node1_1/1 -> butterfly2_1/1(a)
  butterfly2_0/2(a-bw) -> node2_2/1
  butterfly2_0/1(a+bw) -> node2_0/1
  butterfly1_0/4(sync_out) -> butterfly2_0/3(sync)
  node1_2/1 -> butterfly2_0/2(b)
  node1_0/1 -> butterfly2_0/1(a)
  butterfly1_3/3(of) -> of_1/4
  butterfly1_2/3(of) -> of_1/3
  butterfly1_1/3(of) -> of_1/2
  butterfly1_0/3(of) -> of_1/1
  butterfly1_3/2(a-bw) -> node1_7/1
  butterfly1_3/1(a+bw) -> node1_3/1
  node0_7/1 -> butterfly1_3/2(b)
  node0_3/1 -> butterfly1_3/1(a)
  butterfly1_2/2(a-bw) -> node1_6/1
  butterfly1_2/1(a+bw) -> node1_2/1
  node0_6/1 -> butterfly1_2/2(b)
  node0_2/1 -> butterfly1_2/1(a)
  butterfly1_1/2(a-bw) -> node1_5/1
  butterfly1_1/1(a+bw) -> node1_1/1
  node0_5/1 -> butterfly1_1/2(b)
  node0_1/1 -> butterfly1_1/1(a)
  butterfly1_0/2(a-bw) -> node1_4/1
  butterfly1_0/1(a+bw) -> node1_0/1
  node0_4/1 -> butterfly1_0/2(b)
  node0_0/1 -> butterfly1_0/1(a)
  node3_7/1 -> dout_7/1
  node3_6/1 -> dout_3/1
  node3_5/1 -> dout_5/1
  node3_4/1 -> dout_1/1
  node3_3/1 -> dout_6/1
  node3_2/1 -> dout_2/1
  node3_1/1 -> dout_4/1
  node3_0/1 -> dout_0/1
  din_7/1 -> node0_7/1
  din_6/1 -> node0_6/1
  din_5/1 -> node0_5/1
  din_4/1 -> node0_4/1
  din_3/1 -> node0_3/1
  din_2/1 -> node0_2/1
  din_1/1 -> node0_1/1
  din_0/1 -> node0_0/1

== system v01_aa_ri_8r4t11f_ver102/fft_wideband_real1/fft_unscrambler ==
  sync : Inport
  In1 : Inport  Port=2
  In2 : Inport  Port=3
  In3 : Inport  Port=4
  In4 : Inport  Port=5
  const : xbsIndex_r4/Constant  const=1  gui_display_data_type=Fixed-point  arith_type=Boolean  n_bits=1  bin_pt=0  preci_type=Single  exp_width=8  frac_width=24  explicit_period=on  period=1  equ=P=C  opselect=C  inp2=PCIN>>17  opr=+  inp1=P  carry=CIN  dbl_ovrd=off  block_type=constant
  reorder1 : Subsystem
      settings: AttributesFormatString=order=4
  square_transposer1 : Subsystem
      settings: AttributesFormatString=n_inputs=2
  sync_out : Outport
  Out1 : Outport  Port=2
  Out2 : Outport  Port=3
  Out3 : Outport  Port=4
  Out4 : Outport  Port=5
  wires:
  square_transposer1/5(Out4) -> reorder1/6(din3)  "xlsub2_reorder_In4"
  square_transposer1/4(Out3) -> reorder1/5(din2)  "xlsub2_reorder_In3"
  square_transposer1/3(Out2) -> reorder1/4(din1)  "xlsub2_reorder_In2"
  square_transposer1/2(Out1) -> reorder1/3(din0)  "xlsub2_reorder_In1"
  square_transposer1/1(sync_out) -> reorder1/1(sync)
  In4/1 -> square_transposer1/5(In4)
  In3/1 -> square_transposer1/4(In3)
  In2/1 -> square_transposer1/3(In2)
  In1/1 -> square_transposer1/2(In1)
  sync/1 -> square_transposer1/1(sync)
  reorder1/6(dout3) -> Out4/1
  reorder1/5(dout2) -> Out3/1
  reorder1/4(dout1) -> Out2/1
  reorder1/3(dout0) -> Out1/1
  reorder1/1(sync_out) -> sync_out/1
  const/1 -> reorder1/2(en)

== system v01_aa_ri_8r4t11f_ver102/fft_wideband_real1/in_del_4x0pol1 ==
  d : Inport
  q : Outport
  wires:
  d/1 -> q/1

== system v01_aa_ri_8r4t11f_ver102/fft_wideband_real1/in_del_4x0pol2 ==
  d : Inport
  q : Outport
  wires:
  d/1 -> q/1

== system v01_aa_ri_8r4t11f_ver102/fft_wideband_real1/in_del_4x0pol3 ==
  d : Inport
  q : Outport
  wires:
  d/1 -> q/1

== system v01_aa_ri_8r4t11f_ver102/fft_wideband_real1/in_del_4x0pol4 ==
  d : Inport
  q : Outport
  wires:
  d/1 -> q/1

== system v01_aa_ri_8r4t11f_ver102/fft_wideband_real1/in_del_4x1pol1 ==
  d : Inport
  q : Outport
  wires:
  d/1 -> q/1

== system v01_aa_ri_8r4t11f_ver102/fft_wideband_real1/in_del_4x1pol2 ==
  d : Inport
  q : Outport
  wires:
  d/1 -> q/1

== system v01_aa_ri_8r4t11f_ver102/fft_wideband_real1/in_del_4x1pol3 ==
  d : Inport
  q : Outport
  wires:
  d/1 -> q/1

== system v01_aa_ri_8r4t11f_ver102/fft_wideband_real1/in_del_4x1pol4 ==
  d : Inport
  q : Outport
  wires:
  d/1 -> q/1

== system v01_aa_ri_8r4t11f_ver102/fft_wideband_real1/in_del_sync_4x0 ==
  d : Inport
  q : Outport
  wires:
  d/1 -> q/1

== system v01_aa_ri_8r4t11f_ver102/fft_wideband_real1/in_del_sync_4x1 ==
  d : Inport
  q : Outport
  wires:
  d/1 -> q/1

== system v01_aa_ri_8r4t11f_ver102/fft_wideband_real1/out_del_4x0pol1 ==
  d : Inport
  q : Outport
  wires:
  d/1 -> q/1

== system v01_aa_ri_8r4t11f_ver102/fft_wideband_real1/out_del_4x0pol2 ==
  d : Inport
  q : Outport
  wires:
  d/1 -> q/1

== system v01_aa_ri_8r4t11f_ver102/fft_wideband_real1/out_del_4x0pol3 ==
  d : Inport
  q : Outport
  wires:
  d/1 -> q/1

== system v01_aa_ri_8r4t11f_ver102/fft_wideband_real1/out_del_4x0pol4 ==
  d : Inport
  q : Outport
  wires:
  d/1 -> q/1

== system v01_aa_ri_8r4t11f_ver102/fft_wideband_real1/out_del_4x1pol1 ==
  d : Inport
  q : Outport
  wires:
  d/1 -> q/1

== system v01_aa_ri_8r4t11f_ver102/fft_wideband_real1/out_del_4x1pol2 ==
  d : Inport
  q : Outport
  wires:
  d/1 -> q/1

== system v01_aa_ri_8r4t11f_ver102/fft_wideband_real1/out_del_4x1pol3 ==
  d : Inport
  q : Outport
  wires:
  d/1 -> q/1

== system v01_aa_ri_8r4t11f_ver102/fft_wideband_real1/out_del_4x1pol4 ==
  d : Inport
  q : Outport
  wires:
  d/1 -> q/1

== system v01_aa_ri_8r4t11f_ver102/fft_wideband_real1/out_del_sync_4x0 ==
  d : Inport
  q : Outport
  wires:
  d/1 -> q/1
